simulink model "Complete_Model"  (R2023a)
settings: Solver=VariableStepAuto  StartTime=0.0  StopTime=22  MinStep=5e-5  RelTol=1e-3
values from neighbouring files (symbols this model uses):
  Cbus = 80e-3*n_wt_agg*u^2    (= 1.33333333333)    [param.m]
  D = 1e-3    (= 0.001)    [param.m]
  f = 50    [param.m]
  Fs = Vs * sqrt(2/3)/(2*pi*f)    [param.m]
  fsw = 4e3    (= 4000)    [param.m]
  Idr_rated = sqrt(2)*Is    [param.m]
  J = 127*n_wt_agg    (= 19050)    [param.m]
  ki_id = (wni^2)*Lr*sigma    (= 3.27733001332)    [param.m]
  ki_idg = (wnig^2)*Lg    (= 0.263189450696)    [param.m]
  ki_iq = ki_id    (= 3.27733001332)    [param.m]
  ki_iqg = ki_idg    (= 0.263189450696)    [param.m]
  ki_v = -300000*n_wt_agg    (= -45000000)    [param.m]
  Kopt = ((0.5*rho*pi*(Radius^5)*Cp_max)/(lambda_opt^3))    (= 296454.198186)    [param.m]
  kp_id = (2*wni*sigma*Lr) - Rr    (= 0.00384733333333)    [param.m]
  kp_idg = ((2*wnig*Lg)-Rg)    (= 0.00167538274858)    [param.m]
  kp_iq = kp_id    (= 0.00384733333333)    [param.m]
  kp_iqg = kp_idg    (= 0.00167538274858)    [param.m]
  kp_v = -1000*n_wt_agg    (= -150000)    [param.m]
  Kpg = 1/(1.5*Vr*sqrt(2/3))    [param.m]
  Kqg = -Kpg    [param.m]
  lambda_opt = 7.2    [param.m]
  Lg = 400e-6 /n_wt_agg    (= 2.66666666667e-06)    [param.m]
  Lm = 2.5e-3 /n_wt_agg    (= 1.66666666667e-05)    [param.m]
  Lr = Lm + Lsi    (= 1.72466666667e-05)    [param.m]
  Ls = Lm + Lsi    (= 1.72466666667e-05)    [param.m]
  Lsi = 0.087e-3 /n_wt_agg    (= 5.8e-07)    [param.m]
  N = 100    [param.m]
  n_wt_agg = 150    [param.m]
  p = 2    [param.m]
  Ps = 2e6*n_wt_agg    (= 300000000)    [param.m]
  Radius = 42    [param.m]
  Rg = 20e-6 /n_wt_agg    (= 1.33333333333e-07)    [param.m]
  rho = 1.225    [param.m]
  Rr = 2.9e-3 /n_wt_agg    (= 1.93333333333e-05)    [param.m]
  Rs = 2.6e-3 /n_wt_agg    (= 1.73333333333e-05)    [param.m]
  sigma = 1 - Lm^2 / (Ls*Lr)    (= 0.0661284179513)    [param.m]
  smax = 1/3    (= 0.333333333333)    [param.m]
  tab_lambda = [0.1:0.01:11.8]    [param.m]
  Vbus = 1150/u    (= 3450)    [param.m]
  Vs = 690    [param.m]
undefined symbols (not defined in the file or in its folder): Cp, D1, Delta, DiscIntNeverNeededParam, magnitude
libraries: spsSecondOrderFilterLib x133, spsThreePhaseVIMeasurementLib x58, spsPower3phInstantaneousLib x52, simulink x41, spsThreePhaseTransformerTwoWindingsLib x27, spsUniversalBridgeLib x22, spsPWMGenerator2LevelLib x22, spsSeriesRLCBranchLib x19, spsFirstOrderFilterLib x18, spsThreePhasePISectionLineLib x17, spsGroundLib x17, spsThreePhaseSeriesRLCBranchLib x17
(part 10 of 15)

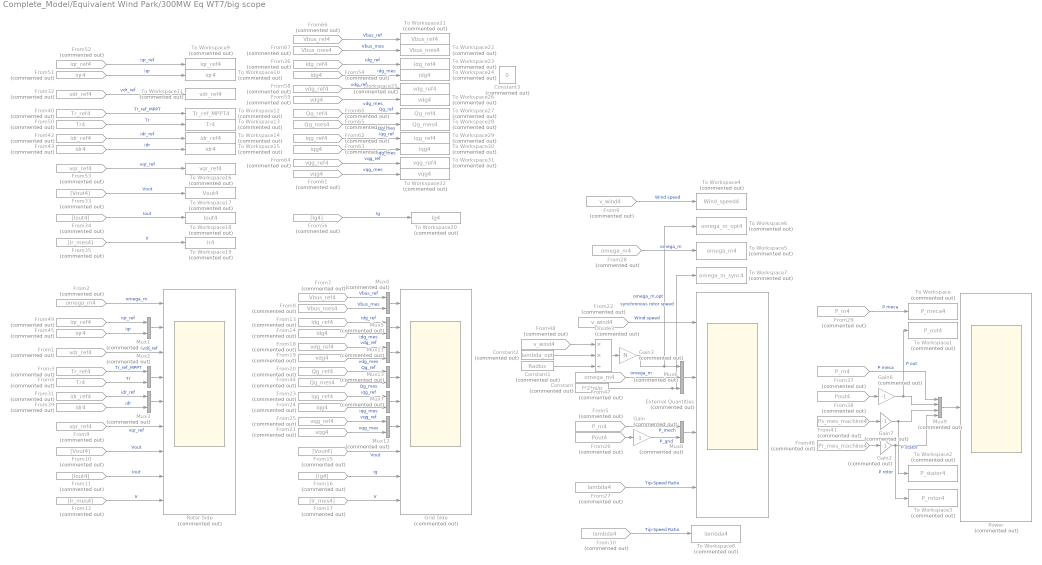
== system Complete_Model/Equivalent Wind Park/300MW Eq WT7/big scope ==
  Constant : Constant  Commented=on  Value=f*2*pi/p
  Constant1 : Constant  Commented=on  Value=Radius
  Constant2 : Constant  Commented=on  Value=lambda_opt
  Constant3 : Constant  Commented=on  Value=0
  Divide3 : Product  Commented=on  Inputs=**/
  External Quantities : Scope  Commented=on  NumInputPorts=4
  From1 : From  Commented=on  GotoTag=vdr_ref4  TagVisibility=global
  From10 : From  Commented=on  GotoTag=Vout4  TagVisibility=global
  From11 : From  Commented=on  GotoTag=Iout4  TagVisibility=global
  From12 : From  Commented=on  GotoTag=Ir_mes4  TagVisibility=global
  From13 : From  Commented=on  GotoTag=idg_ref4  TagVisibility=global
  From14 : From  Commented=on  GotoTag=idg4  TagVisibility=global
  From15 : From  Commented=on  GotoTag=Vout4  TagVisibility=global
  From16 : From  Commented=on  GotoTag=Ig4  TagVisibility=global
  From17 : From  Commented=on  GotoTag=Ir_mes4  TagVisibility=global
  From18 : From  Commented=on  GotoTag=vdg_ref4  TagVisibility=global
  From19 : From  Commented=on  GotoTag=vdg4  TagVisibility=global
  From2 : From  Commented=on  GotoTag=omega_m4  TagVisibility=global
  From20 : From  Commented=on  GotoTag=Qg_ref4  TagVisibility=global
  From21 : From  Commented=on  GotoTag=vqg4  TagVisibility=global
  From22 : From  Commented=on  GotoTag=v_wind4  TagVisibility=global
  From23 : From  Commented=on  GotoTag=iqg_ref4  TagVisibility=global
  From24 : From  Commented=on  GotoTag=iqg4  TagVisibility=global
  From25 : From  Commented=on  GotoTag=vqg_ref4  TagVisibility=global
  From26 : From  Commented=on  GotoTag=Pout4  TagVisibility=global
  From27 : From  Commented=on  GotoTag=lambda4  TagVisibility=global
  From28 : From  Commented=on  GotoTag=omega_m4  TagVisibility=global
  From29 : From  Commented=on  GotoTag=P_m4  TagVisibility=global
  From3 : From  Commented=on  GotoTag=Tr_ref4  TagVisibility=global
  From30 : From  Commented=on  GotoTag=lambda4  TagVisibility=global
  From31 : From  Commented=on  GotoTag=idr_ref4  TagVisibility=global
  From32 : From  Commented=on  GotoTag=vdr_ref4  TagVisibility=global
  From33 : From  Commented=on  GotoTag=Vout4  TagVisibility=global
  From34 : From  Commented=on  GotoTag=Iout4  TagVisibility=global
  From35 : From  Commented=on  GotoTag=Ir_mes4  TagVisibility=global
  From36 : From  Commented=on  GotoTag=idg_ref4  TagVisibility=global
  From37 : From  Commented=on  GotoTag=P_m4  TagVisibility=global
  From38 : From  Commented=on  GotoTag=Pout4  TagVisibility=global
  From39 : From  Commented=on  GotoTag=idr4  TagVisibility=global
  From4 : From  Commented=on  GotoTag=Tr4  TagVisibility=global
  From40 : From  Commented=on  GotoTag=Tr_ref4  TagVisibility=global
  From41 : From  Commented=on  GotoTag=Ps_mes_machine4  TagVisibility=global
  From42 : From  Commented=on  GotoTag=idr_ref4  TagVisibility=global
  From43 : From  Commented=on  GotoTag=idr4  TagVisibility=global
  From44 : From  Commented=on  GotoTag=Qg_mes4  TagVisibility=global
  From45 : From  Commented=on  GotoTag=iqr4  TagVisibility=global
  From46 : From  Commented=on  GotoTag=Pr_mes_machine4  TagVisibility=global
  From47 : From  Commented=on  GotoTag=omega_m4  TagVisibility=global
  From48 : From  Commented=on  GotoTag=v_wind4  TagVisibility=global
  From49 : From  Commented=on  GotoTag=iqr_ref4  TagVisibility=global
  From5 : From  Commented=on  GotoTag=P_m4  TagVisibility=global
  From50 : From  Commented=on  GotoTag=Tr4  TagVisibility=global
  From51 : From  Commented=on  GotoTag=iqr4  TagVisibility=global
  From52 : From  Commented=on  GotoTag=iqr_ref4  TagVisibility=global
  From53 : From  Commented=on  GotoTag=vqr_ref4  TagVisibility=global
  From54 : From  Commented=on  GotoTag=idg4  TagVisibility=global
  From56 : From  Commented=on  GotoTag=Ig4  TagVisibility=global
  From58 : From  Commented=on  GotoTag=vdg_ref4  TagVisibility=global
  From59 : From  Commented=on  GotoTag=vdg4  TagVisibility=global
  From6 : From  Commented=on  GotoTag=v_wind4  TagVisibility=global
  From60 : From  Commented=on  GotoTag=Qg_ref4  TagVisibility=global
  From61 : From  Commented=on  GotoTag=vqg4  TagVisibility=global
  From62 : From  Commented=on  GotoTag=iqg_ref4  TagVisibility=global
  From63 : From  Commented=on  GotoTag=iqg4  TagVisibility=global
  From64 : From  Commented=on  GotoTag=vqg_ref4  TagVisibility=global
  From65 : From  Commented=on  GotoTag=Qg_mes4  TagVisibility=global
  From66 : From  Commented=on  GotoTag=Vbus_ref4  TagVisibility=global
  From67 : From  Commented=on  GotoTag=Vbus_mes4  TagVisibility=global
  From7 : From  Commented=on  GotoTag=Vbus_ref4  TagVisibility=global
  From8 : From  Commented=on  GotoTag=Vbus_mes4  TagVisibility=global
  From9 : From  Commented=on  GotoTag=vqr_ref4  TagVisibility=global
  Gain{,2,6..7} (4 alike) : Gain  Commented=on  Gain=-1
  Gain3 : Gain  Commented=on  Gain=N
  Grid Side : Scope  Commented=on  NumInputPorts=9
  Mux{1..5,7..8,11..13} (10 alike) : Mux  Commented=on  Inputs=2
  Mux6 : Mux  Commented=on  Inputs=3
  Mux9 : Mux  Commented=on
  Power : Scope  Commented=on
  Rotor Side : Scope  Commented=on  NumInputPorts=9
  To Workspace : ToWorkspace  Commented=on  VariableName=P_meca4  SaveFormat=Timeseries  SampleTime=-1
  To Workspace1 : ToWorkspace  Commented=on  VariableName=P_out4  SaveFormat=Timeseries  SampleTime=-1
  To Workspace10 : ToWorkspace  Commented=on  VariableName=iqr4  SaveFormat=Timeseries  SampleTime=-1
  To Workspace11 : ToWorkspace  Commented=on  VariableName=vdr_ref4  SaveFormat=Timeseries  SampleTime=-1
  To Workspace12 : ToWorkspace  Commented=on  VariableName=Tr_ref_MPPT4  SaveFormat=Timeseries  SampleTime=-1
  To Workspace13 : ToWorkspace  Commented=on  VariableName=Tr4  SaveFormat=Timeseries  SampleTime=-1
  To Workspace14 : ToWorkspace  Commented=on  VariableName=idr_ref4  SaveFormat=Timeseries  SampleTime=-1
  To Workspace15 : ToWorkspace  Commented=on  VariableName=idr4  SaveFormat=Timeseries  SampleTime=-1
  To Workspace16 : ToWorkspace  Commented=on  VariableName=vqr_ref4  SaveFormat=Timeseries  SampleTime=-1
  To Workspace17 : ToWorkspace  Commented=on  VariableName=Vout4  SaveFormat=Timeseries  SampleTime=-1
  To Workspace18 : ToWorkspace  Commented=on  VariableName=Iout4  SaveFormat=Timeseries  SampleTime=-1
  To Workspace19 : ToWorkspace  Commented=on  VariableName=Ir4  SaveFormat=Timeseries  SampleTime=-1
  To Workspace2 : ToWorkspace  Commented=on  VariableName=P_stator4  SaveFormat=Timeseries  SampleTime=-1
  To Workspace20 : ToWorkspace  Commented=on  VariableName=Ig4  SaveFormat=Timeseries  SampleTime=-1
  To Workspace21 : ToWorkspace  Commented=on  VariableName=Vbus_ref4  SaveFormat=Timeseries  SampleTime=-1
  To Workspace22 : ToWorkspace  Commented=on  VariableName=Vbus_mes4  SaveFormat=Timeseries  SampleTime=-1
  To Workspace23 : ToWorkspace  Commented=on  VariableName=idg_ref4  SaveFormat=Timeseries  SampleTime=-1
  To Workspace24 : ToWorkspace  Commented=on  VariableName=idg4  SaveFormat=Timeseries  SampleTime=-1
  To Workspace25 : ToWorkspace  Commented=on  VariableName=vdg_ref4  SaveFormat=Timeseries  SampleTime=-1
  To Workspace26 : ToWorkspace  Commented=on  VariableName=vdg4  SaveFormat=Timeseries  SampleTime=-1
  To Workspace27 : ToWorkspace  Commented=on  VariableName=Qg_ref4  SaveFormat=Timeseries  SampleTime=-1
  To Workspace28 : ToWorkspace  Commented=on  VariableName=Qg_mes4  SaveFormat=Timeseries  SampleTime=-1
  To Workspace29 : ToWorkspace  Commented=on  VariableName=iqg_ref4  SaveFormat=Timeseries  SampleTime=-1
  To Workspace3 : ToWorkspace  Commented=on  VariableName=P_rotor4  SaveFormat=Timeseries  SampleTime=-1
  To Workspace30 : ToWorkspace  Commented=on  VariableName=iqg4  SaveFormat=Timeseries  SampleTime=-1
  To Workspace31 : ToWorkspace  Commented=on  VariableName=vqg_ref4  SaveFormat=Timeseries  SampleTime=-1
  To Workspace32 : ToWorkspace  Commented=on  VariableName=vqg4  SaveFormat=Timeseries  SampleTime=-1
  To Workspace4 : ToWorkspace  Commented=on  VariableName=Wind_speed4  SaveFormat=Timeseries  SampleTime=-1
  To Workspace5 : ToWorkspace  Commented=on  VariableName=omega_m4  SaveFormat=Timeseries  SampleTime=-1
  To Workspace6 : ToWorkspace  Commented=on  VariableName=omega_m_opt4  SaveFormat=Timeseries  SampleTime=-1
  To Workspace7 : ToWorkspace  Commented=on  VariableName=omega_m_sync4  SaveFormat=Timeseries  SampleTime=-1
  To Workspace8 : ToWorkspace  Commented=on  VariableName=lambda4  SaveFormat=Timeseries  SampleTime=-1
  To Workspace9 : ToWorkspace  Commented=on  VariableName=iqr_ref4  SaveFormat=Timeseries  SampleTime=-1
  wires:
  From61/1 -> To Workspace32/1  "vqg_mes"
  From64/1 -> To Workspace31/1  "vqg_ref"
  From63/1 -> To Workspace30/1  "iqg_mes"
  From62/1 -> To Workspace29/1  "iqg_ref"
  From65/1 -> To Workspace28/1  "Qg_mes"
  From59/1 -> To Workspace26/1  "vdg_mes"
  From60/1 -> To Workspace27/1  "Qg_ref"
  From58/1 -> To Workspace25/1  "vdg_ref"
  From54/1 -> To Workspace24/1  "idg_mes"
  From36/1 -> To Workspace23/1  "idg_ref"
  From67/1 -> To Workspace22/1  "Vbus_mes"
  From66/1 -> To Workspace21/1  "Vbus_ref"
  From56/1 -> To Workspace20/1  "Ig"
  From35/1 -> To Workspace19/1  "Ir"
  From34/1 -> To Workspace18/1  "Iout"
  From33/1 -> To Workspace17/1  "Vout"
  From53/1 -> To Workspace16/1  "vqr_ref"
  From43/1 -> To Workspace15/1  "idr"
  From42/1 -> To Workspace14/1  "idr_ref"
  From50/1 -> To Workspace13/1  "Tr"
  From40/1 -> To Workspace12/1  "Tr_ref_MPPT"
  From32/1 -> To Workspace11/1  "vdr_ref"
  From51/1 -> To Workspace10/1  "iqr"
  From52/1 -> To Workspace9/1  "iqr_ref"
  From30/1 -> To Workspace8/1  "Tip-Speed Ratio"
  From29/1 -> To Workspace/1  "P meca"
  From28/1 -> To Workspace5/1  "omega_m"
  From6/1 -> To Workspace4/1  "Wind speed"
  From27/1 -> External Quantities/4  "Tip-Speed Ratio"
  Gain3/1 -> Mux6/1, To Workspace6/1  "omega_m,opt"
  Divide3/1 -> Gain3/1
  Constant1/1 -> Divide3/3
  Constant2/1 -> Divide3/2
  From48/1 -> Divide3/1
  From39/1 -> Mux3/2  "idr"
  From4/1 -> Mux2/2  "Tr"
  From1/1 -> Rotor Side/3  "vdr_ref"
  From49/1 -> Mux1/1  "iqr_ref"
  Mux13/1 -> Grid Side/4
  From44/1 -> Mux13/2  "Qg_mes"
  From20/1 -> Mux13/1  "Qg_ref"
  Mux12/1 -> Grid Side/6
  From25/1 -> Mux12/1  "vqg_ref"
  From21/1 -> Mux12/2  "vqg_mes"
  Gain2/1 -> Mux9/4, To Workspace3/1  "P rotor"
  From38/1 -> Gain6/1
  Mux9/1 -> Power/1
  From37/1 -> Mux9/1  "P meca"
  From46/1 -> Gain2/1
  Gain7/1 -> Mux9/3, To Workspace2/1  "P stator"
  From41/1 -> Gain7/1
  Gain6/1 -> Mux9/2, To Workspace1/1  "P out"
  Mux11/1 -> Grid Side/3
  From19/1 -> Mux11/2  "vdg_mes"
  From18/1 -> Mux11/1  "vdg_ref"
  From5/1 -> Mux8/1  "P_mech"
  Constant/1 -> Mux6/3, To Workspace7/1  "synchronous rotor speed"
  From47/1 -> Mux6/2  "omega_m"
  From22/1 -> External Quantities/1  "Wind speed"
  Mux6/1 -> External Quantities/2
  Mux8/1 -> External Quantities/3
  From26/1 -> Gain/1
  Gain/1 -> Mux8/2  "P_grid"
  From2/1 -> Rotor Side/1  "omega_m"
  From13/1 -> Mux5/1  "idg_ref"
  Mux4/1 -> Grid Side/1
  From14/1 -> Mux5/2  "idg_mes"
  Mux5/1 -> Grid Side/2
  From8/1 -> Mux4/2  "Vbus_mes"
  Mux7/1 -> Grid Side/5
  From7/1 -> Mux4/1  "Vbus_ref"
  From24/1 -> Mux7/2  "iqg_mes"
  From17/1 -> Grid Side/9  "Ir"
  From15/1 -> Grid Side/7  "Vout"
  From23/1 -> Mux7/1  "iqg_ref"
  From16/1 -> Grid Side/8  "Ig"
  From12/1 -> Rotor Side/9  "Ir"
  From11/1 -> Rotor Side/8  "Iout"
  From10/1 -> Rotor Side/7  "Vout"
  From9/1 -> Rotor Side/6  "vqr_ref"
  From31/1 -> Mux3/1  "idr_ref"
  Mux3/1 -> Rotor Side/5
  Mux2/1 -> Rotor Side/4
  From3/1 -> Mux2/1  "Tr_ref_MPPT"
  Mux1/1 -> Rotor Side/2
  From45/1 -> Mux1/2  "iqr"

== system Complete_Model/Equivalent Wind Park/300MW Eq WT8/Aerodynamic Model ==
  note: Gearbox ratio
  note: Motor convention : Generated power
      |  is negative
  note: \Omega_m blades
  note: Cp
  note: \lambda = \Omega_m R / v_{wind}
  note: C_p (\lambda)
  note: v_{wind}
  Vw : Inport
  C_p (\lambda) : Lookup_n-D  NumberOfTableDimensions=1  BreakpointsForDimension1=tab_lambda  Table=Cp  InternalRulePriority=Speed  RndMeth=Simplest
  Constant : Constant  Value=Radius
  Divide : Product  Inputs=*/
  Divide1 : Product  Inputs=**/
  Fcn2 : Fcn  Expr=0.5*rho*pi*Radius^2*u(2)^3*u(1)
  Gain : Gain  Gain=1/N
  Gain2 : Gain  Gain=n_wt_agg
  Gain4 : Gain  Gain=-1
  Mux2 : Mux  Inputs=2
  Tm : Outport
  tag scope: P_m11=global, Tm11=global, lambda11=global
  wires:
  Gain4/1 -> Divide/1
  Constant/1 -> Divide1/1
  Divide/1 -> Tm/1, [Tm11]
  [omega_m11] -> Divide/2
  Gain2/1 -> Gain4/1, [P_m11]
  Fcn2/1 -> Gain2/1
  Mux2/1 -> Fcn2/1
  C_p (\lambda)/1 -> Mux2/1
  Divide1/1 -> [lambda11], C_p (\lambda)/1  "lambda"
  Vw/1 -> Mux2/2, Divide1/3
  Gain/1 -> Divide1/2
  [omega_m11] -> Gain/1

== system Complete_Model/Equivalent Wind Park/300MW Eq WT8/Grid Side Control ==
  Vbus_ref : Inport
  Qg_ref : Inport  Port=2
  Vbus : Inport  Port=3
  Ig_mes : Inport  Port=4
  Vs_mes : Inport  Port=5
  Demux : Demux  Outputs=2
  Demux1 : Demux  Outputs=2
  Gain : Gain  Gain=Kqg
  Gain1 : Gain  Gain=Kpg
  Normalized for PWM : Gain  Gain=1/(Vbus/2)
  "PI " : Subsystem
  PI id : Subsystem
  PI iq : Subsystem
  Sum : Sum  Inputs=|++
  Vs_mes1 : InportShadow  Port=5
  abc->alpha/beta : Subsystem
  abc->alpha/beta1 : Subsystem
  alpha/beta->abc : Subsystem
  alpha/beta->d/q : Subsystem
  alpha/beta->d/q1 : Subsystem
  angle2 : Subsystem
  cancellation of coupling terms : Subsystem
  d/q->alpha/beta : Subsystem
  Vabc_ref : Outport
  tag scope: Qg_ref11=global, Vbus_ref11=global, idg11=global, idg_ref11=global, iqg11=global, iqg_ref11=global, vdg11=global, vqg11=global
  wires:
  Vs_mes1/1 -> Sum/2
  angle2/1(theta_g) -> [theta_grid11]
  [theta_grid11] -> alpha/beta->d/q1/2(theta)
  [theta_grid11] -> d/q->alpha/beta/2(theta)
  [theta_grid11] -> alpha/beta->d/q/2(theta)
  Demux/2 -> PI iq/2(meas), [iqg11]
  Demux/1 -> PI id/2(meas), [idg11]
  Vbus/1 -> "PI "/2(meas)
  Demux1/1 -> [vdg11]
  Demux1/2 -> [vqg11]
  alpha/beta->d/q1/1(d/q) -> Demux1/1
  abc->alpha/beta1/1(alpha/beta) -> alpha/beta->d/q1/1(D/Q)
  [Vg11] -> abc->alpha/beta1/1(abc)
  cancellation of coupling terms/1(out) -> d/q->alpha/beta/1(d/q)
  Sum/1 -> Normalized for PWM/1
  alpha/beta->abc/1(abc) -> Sum/1
  Qg_ref/1 -> Gain/1, [Qg_ref11]
  Gain1/1 -> PI id/1(ref), [idg_ref11]  "idg"
  "PI "/1(out) -> Gain1/1  "Pg_ref"
  Gain/1 -> [iqg_ref11], PI iq/1(ref)  "iqg"
  Vbus_ref/1 -> "PI "/1(ref), [Vbus_ref11]
  alpha/beta->d/q/1(d/q) -> Demux/1
  PI iq/1(out) -> cancellation of coupling terms/2(Vq*)
  PI id/1(out) -> cancellation of coupling terms/1(Vd*)
  Vs_mes/1 -> angle2/1(Vs_mes)
  Normalized for PWM/1 -> Vabc_ref/1
  abc->alpha/beta/1(alpha/beta) -> alpha/beta->d/q/1(D/Q)
  Ig_mes/1 -> abc->alpha/beta/1(abc)
  d/q->alpha/beta/1(D/Q) -> alpha/beta->abc/1(D/Q)

== system Complete_Model/Equivalent Wind Park/300MW Eq WT8/Power Calculations ==
  Demux2 : Demux  Outputs=2
  Demux3 : Demux  Outputs=2
  Power (3ph, Instantaneous){1..4} (4 alike) : spsPower3phInstantaneousLib/Power (3ph, Instantaneous)
  Scope{1,3..5,10..13} (8 alike) : Scope
  "Second-Order " : spsSecondOrderFilterLib/Second-Order Filter  FilterType=Lowpass  Fo=17  Zeta=0.7  Ts=7.4074e-05  Initialize=off  Vac_Init="[0,  0,  60]"  Vdc_Init=0  PlotResponse=off  FreqRange=[0, 500, 1]
      settings: AttributesFormatString=f0=%<Fo>
  Second-Order Low-Pass Filter {1..7} (7 alike) : spsSecondOrderFilterLib/Second-Order Filter  FilterType=Lowpass  Fo=17  Zeta=0.7  Ts=7.4074e-05  Initialize=off  Vac_Init="[0,  0,  60]"  Vdc_Init=0  PlotResponse=off  FreqRange=[0, 500, 1]
      settings: AttributesFormatString=f0=%<Fo>
  Subsystem2 : Subsystem
  Subsystem3 : Subsystem
  To Workspace2 : ToWorkspace  VariableName=Ig_d11  SaveFormat=Timeseries  SampleTime=-1
  To Workspace3 : ToWorkspace  VariableName=Vg_d11  SaveFormat=Timeseries  SampleTime=-1
  ToFile_Ig_d11 : ToFile  Filename=Ig_d11.mat  SaveFormat=Timeseries
  ToFile_Vg_d11 : ToFile  Filename=Vg_d11.mat  SaveFormat=Timeseries
  alpha/beta->d/q2 : Subsystem
  alpha/beta->d/q3 : Subsystem
  angle1 : Subsystem
  tag scope: Pg_mes11=global, Pout11=global, Pr_mes_machine11=global, Ps_mes_machine11=global, Qg_mes11=global, Qout11=global, Qr_mes_machine11=global, Qs_mes_machine11=global
  wires:
  [Vg11] -> angle1/1(Vs_mes), Subsystem2/1(abc)
  Subsystem2/1(alpha/beta) -> alpha/beta->d/q2/1(D/Q)
  alpha/beta->d/q2/1(d/q) -> Demux2/1
  Demux2/1 -> ToFile_Vg_d11/1, Scope10/1, To Workspace3/1  "Vg_d11"
  Demux2/2 -> Scope11/1
  Demux3/2 -> Scope13/1
  Demux3/1 -> ToFile_Ig_d11/1, Scope12/1, To Workspace2/1  "Ig_d11"
  alpha/beta->d/q3/1(d/q) -> Demux3/1
  Subsystem3/1(alpha/beta) -> alpha/beta->d/q3/1(D/Q)
  angle1/1(theta_g) -> alpha/beta->d/q3/2(theta), alpha/beta->d/q2/2(theta)
  [Ig11] -> Subsystem3/1(abc)
  [Vr_mes11] -> "Power (3ph, Instantaneous)4"/1
  "Power (3ph, Instantaneous)1"/1 -> Second-Order Low-Pass Filter 7/1  "Pr"
  "Power (3ph, Instantaneous)4"/1 -> Second-Order Low-Pass Filter 5/1  "Pr"
  [Vout11] -> "Power (3ph, Instantaneous)2"/1
  "Power (3ph, Instantaneous)3"/2 -> Second-Order Low-Pass Filter 2/1
  [Vg11] -> "Power (3ph, Instantaneous)1"/1
  Second-Order Low-Pass Filter 2/1 -> [Qs_mes_machine11]  "Q_stator"
  [Vs_mes_machine11] -> "Power (3ph, Instantaneous)3"/1
  "Power (3ph, Instantaneous)2"/1 -> "Second-Order "/1
  Second-Order Low-Pass Filter 5/1 -> [Pr_mes_machine11]  "P_rotor"
  [Is_mes_machine11] -> "Power (3ph, Instantaneous)3"/2, Scope4/1
  Second-Order Low-Pass Filter 7/1 -> [Pg_mes11]  "P_rotor"
  [Ir_mes11] -> "Power (3ph, Instantaneous)4"/2, Scope5/1
  "Second-Order "/1 -> [Pout11]  "Pout"
  Second-Order Low-Pass Filter 6/1 -> [Qg_mes11]  "Q_rotor"
  Second-Order Low-Pass Filter 4/1 -> [Qr_mes_machine11]  "Q_rotor"
  "Power (3ph, Instantaneous)3"/1 -> Second-Order Low-Pass Filter 3/1  "Ps"
  [Ig11] -> Scope1/1, "Power (3ph, Instantaneous)1"/2
  "Power (3ph, Instantaneous)4"/2 -> Second-Order Low-Pass Filter 4/1
  [Iout11] -> "Power (3ph, Instantaneous)2"/2, Scope3/1
  "Power (3ph, Instantaneous)1"/2 -> Second-Order Low-Pass Filter 6/1
  Second-Order Low-Pass Filter 3/1 -> [Ps_mes_machine11]  "P_stator"
  Second-Order Low-Pass Filter 1/1 -> [Qout11]  "Qout"
  "Power (3ph, Instantaneous)2"/2 -> Second-Order Low-Pass Filter 1/1

== system Complete_Model/Equivalent Wind Park/300MW Eq WT8/Rotor Side Control ==
  note: Stator referred
  idr_ref : Inport
  Ir_mes : Inport  Port=2
  Vs_mes : Inport  Port=3
  theta_m_mes : Inport  Port=4
  speed_mes : Inport  Port=5
  D/Q->abc : Subsystem
  D/Q->d/q : Subsystem
  Demux : Demux  Outputs=2
  Gain : Gain  Gain=1/(-1.5*p*(Lm/Ls)*Fs)
  Gain1 : Gain  Gain=1/u
  Gain2 : Gain  Gain=1/u
  MPPT : Subsystem
  Normalized for PWM : Gain  Gain=1/(Vbus/2)
  PI id : Subsystem
  PI iq : Subsystem
  abc->alpha/beta : Subsystem
  angle : Subsystem
  cancellation of coupling terms : Subsystem
  d/q->D/Q : Subsystem
  Vabc_ref : Outport
  tag scope: Tr_ref11=global, idr11=global, idr_ref11=global, iqr11=global, iqr_ref11=global, vrabc_ref11=global
  wires:
  MPPT/1(Tm,opt) -> Gain/1, [Tr_ref11]
  d/q->D/Q/1(D/Q) -> D/Q->abc/1(D/Q)
  abc->alpha/beta/1(alpha/beta) -> D/Q->d/q/1(D/Q)
  Gain2/1 -> [vrabc_ref11], Normalized for PWM/1  "Rotor referred"
  D/Q->abc/1(abc) -> Gain2/1
  Normalized for PWM/1 -> Vabc_ref/1
  Gain1/1 -> abc->alpha/beta/1(abc)
  idr_ref/1 -> PI id/1(ref), [idr_ref11]
  Gain/1 -> PI iq/1(ref), [iqr_ref11]
  Demux/2 -> PI iq/2(meas), [iqr11]
  Demux/1 -> [idr11], PI id/2(meas)
  D/Q->d/q/1(d/q) -> Demux/1  "i_dq = rotor ref frame"
  speed_mes/1 -> MPPT/1(omega_m), cancellation of coupling terms/3(omega_m)
  cancellation of coupling terms/1(out) -> d/q->D/Q/1(d/q)  "Vdr_ref,Vqr_ref"
  PI iq/1(out) -> cancellation of coupling terms/2(Vq*)
  PI id/1(out) -> cancellation of coupling terms/1(Vd*)
  angle/1(theta_r) -> D/Q->d/q/2(theta), d/q->D/Q/2(theta)
  Vs_mes/1 -> angle/1(Vs_mes)
  theta_m_mes/1 -> angle/2(theta_m)
  Ir_mes/1 -> Gain1/1

== system Complete_Model/Equivalent Wind Park/300MW Eq WT8/Subsystem ==
  In1 : Inport
  Bus Selector : BusSelector  OutputSignals=Mechanical.Rotor angle thetam (rad),Mechanical.Rotor speed (wm),Mechanical.Electromagnetic torque Te (N*m)
  Second-Order Low-Pass Filter 3 : spsSecondOrderFilterLib/Second-Order Filter  FilterType=Lowpass  Fo=17  Zeta=0.7  Ts=7.4074e-05  Initialize=off  Vac_Init="[0,  0,  60]"  Vdc_Init=0  PlotResponse=off  FreqRange=[0, 500, 1]
      settings: AttributesFormatString=f0=%<Fo>
  tag scope: Tr11=global, omega_m11=global, theta11=global
  wires:
  Second-Order Low-Pass Filter 3/1 -> [omega_m11]
  Bus Selector/3 -> [Tr11]  "<Electromagnetic torque Te (N*m)>"
  Bus Selector/1 -> [theta11]  "<Rotor angle thetam (rad)>"
  In1/1 -> Bus Selector/1
  Bus Selector/2 -> Second-Order Low-Pass Filter 3/1  "<Rotor speed (wm)>"

== system Complete_Model/Equivalent Wind Park/300MW Eq WT8/Subsystem1 ==
  Constant4 : Constant  Value=0
  Step : Step  Time=50  After=-0.2*Ps  SampleTime=0
  Sum14 : Sum  Inputs=++|
  Out1 : Outport
  wires:
  Sum14/1 -> Out1/1
  Step/1 -> Sum14/1
  Constant4/1 -> Sum14/2

== system Complete_Model/Equivalent Wind Park/300MW Eq WT8/Subsystem2 ==
  Constant1 : Constant  Commented=on  Value=8
  Constant2 : Constant  Value=9
  Ramp1 : simulink/Sources/Ramp  Commented=on  slope=-1  start=16  InitialOutput=0
  Ramp2 : simulink/Sources/Ramp  Commented=on  slope=1  start=13  InitialOutput=0
  Ramp3 : simulink/Sources/Ramp  Commented=on  slope=-1  start=36  InitialOutput=0
  Ramp4 : simulink/Sources/Ramp  Commented=on  slope=1  start=35  InitialOutput=0
  Ramp5 : simulink/Sources/Ramp  slope=-0.4  start=13  InitialOutput=0
  Ramp6 : simulink/Sources/Ramp  slope=0.4  start=8  InitialOutput=0
  Sum{1..4} (4 alike) : Sum  Commented=on  Inputs=++|
  Sum5 : Sum  Inputs=++|
  Sum6 : Sum  Inputs=++|
  v_wind : Outport
  tag scope: v_wind11=global
  wires:
  Sum4/1 -> ?
  Ramp2/1 -> Sum1/1
  Ramp1/1 -> Sum1/2
  Sum1/1 -> Sum2/1
  Sum3/1 -> Sum4/1
  Ramp3/1 -> Sum3/2
  Ramp4/1 -> Sum3/1
  Sum2/1 -> Sum4/2
  Constant1/1 -> Sum2/2
  Ramp5/1 -> Sum5/2
  Constant2/1 -> Sum6/2
  Ramp6/1 -> Sum5/1
  Sum5/1 -> Sum6/1
  Sum6/1 -> v_wind/1, [v_wind11]

== system Complete_Model/Equivalent Wind Park/300MW Eq WT8/Subsystem8 ==
  Constant4 : Constant  Value=0
  Step : Step  Time=55  After=Idr_rated*0.2  SampleTime=0
  Sum14 : Sum  Inputs=++|
  Out1 : Outport
  wires:
  Sum14/1 -> Out1/1
  Constant4/1 -> Sum14/2
  Step/1 -> Sum14/1

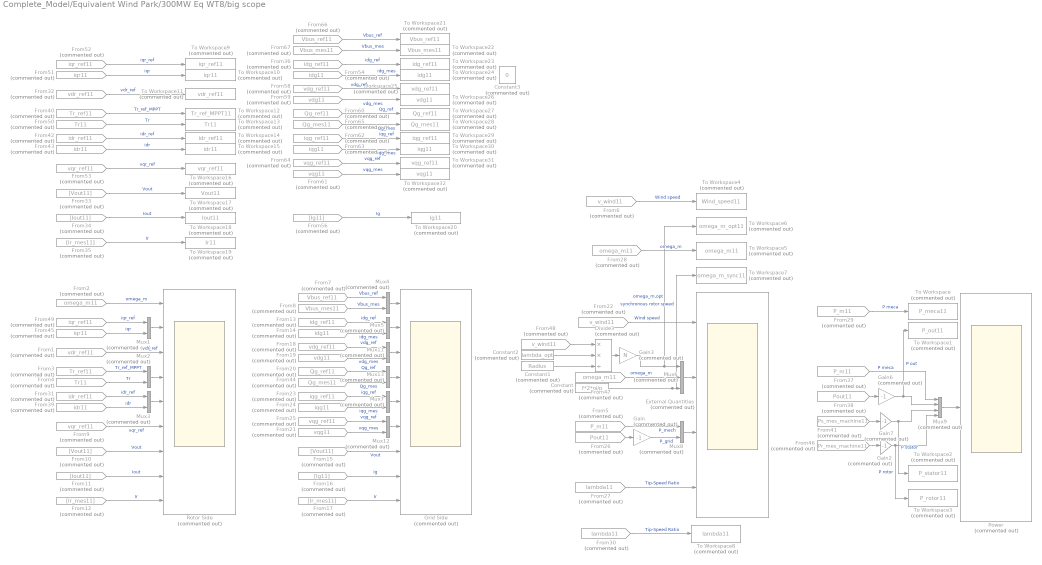
== system Complete_Model/Equivalent Wind Park/300MW Eq WT8/big scope ==
  Constant : Constant  Commented=on  Value=f*2*pi/p
  Constant1 : Constant  Commented=on  Value=Radius
  Constant2 : Constant  Commented=on  Value=lambda_opt
  Constant3 : Constant  Commented=on  Value=0
  Divide3 : Product  Commented=on  Inputs=**/
  External Quantities : Scope  Commented=on  NumInputPorts=4
  From1 : From  Commented=on  GotoTag=vdr_ref11  TagVisibility=global
  From10 : From  Commented=on  GotoTag=Vout11  TagVisibility=global
  From11 : From  Commented=on  GotoTag=Iout11  TagVisibility=global
  From12 : From  Commented=on  GotoTag=Ir_mes11  TagVisibility=global
  From13 : From  Commented=on  GotoTag=idg_ref11  TagVisibility=global
  From14 : From  Commented=on  GotoTag=idg11  TagVisibility=global
  From15 : From  Commented=on  GotoTag=Vout11  TagVisibility=global
  From16 : From  Commented=on  GotoTag=Ig11  TagVisibility=global
  From17 : From  Commented=on  GotoTag=Ir_mes11  TagVisibility=global
  From18 : From  Commented=on  GotoTag=vdg_ref11  TagVisibility=global
  From19 : From  Commented=on  GotoTag=vdg11  TagVisibility=global
  From2 : From  Commented=on  GotoTag=omega_m11  TagVisibility=global
  From20 : From  Commented=on  GotoTag=Qg_ref11  TagVisibility=global
  From21 : From  Commented=on  GotoTag=vqg11  TagVisibility=global
  From22 : From  Commented=on  GotoTag=v_wind11  TagVisibility=global
  From23 : From  Commented=on  GotoTag=iqg_ref11  TagVisibility=global
  From24 : From  Commented=on  GotoTag=iqg11  TagVisibility=global
  From25 : From  Commented=on  GotoTag=vqg_ref11  TagVisibility=global
  From26 : From  Commented=on  GotoTag=Pout11  TagVisibility=global
  From27 : From  Commented=on  GotoTag=lambda11  TagVisibility=global
  From28 : From  Commented=on  GotoTag=omega_m11  TagVisibility=global
  From29 : From  Commented=on  GotoTag=P_m11  TagVisibility=global
  From3 : From  Commented=on  GotoTag=Tr_ref11  TagVisibility=global
  From30 : From  Commented=on  GotoTag=lambda11  TagVisibility=global
  From31 : From  Commented=on  GotoTag=idr_ref11  TagVisibility=global
  From32 : From  Commented=on  GotoTag=vdr_ref11  TagVisibility=global
  From33 : From  Commented=on  GotoTag=Vout11  TagVisibility=global
  From34 : From  Commented=on  GotoTag=Iout11  TagVisibility=global
  From35 : From  Commented=on  GotoTag=Ir_mes11  TagVisibility=global
  From36 : From  Commented=on  GotoTag=idg_ref11  TagVisibility=global
  From37 : From  Commented=on  GotoTag=P_m11  TagVisibility=global
  From38 : From  Commented=on  GotoTag=Pout11  TagVisibility=global
  From39 : From  Commented=on  GotoTag=idr11  TagVisibility=global
  From4 : From  Commented=on  GotoTag=Tr11  TagVisibility=global
  From40 : From  Commented=on  GotoTag=Tr_ref11  TagVisibility=global
  From41 : From  Commented=on  GotoTag=Ps_mes_machine11  TagVisibility=global
  From42 : From  Commented=on  GotoTag=idr_ref11  TagVisibility=global
  From43 : From  Commented=on  GotoTag=idr11  TagVisibility=global
  From44 : From  Commented=on  GotoTag=Qg_mes11  TagVisibility=global
  From45 : From  Commented=on  GotoTag=iqr11  TagVisibility=global
  From46 : From  Commented=on  GotoTag=Pr_mes_machine11  TagVisibility=global
  From47 : From  Commented=on  GotoTag=omega_m11  TagVisibility=global
  From48 : From  Commented=on  GotoTag=v_wind11  TagVisibility=global
  From49 : From  Commented=on  GotoTag=iqr_ref11  TagVisibility=global
  From5 : From  Commented=on  GotoTag=P_m11  TagVisibility=global
  From50 : From  Commented=on  GotoTag=Tr11  TagVisibility=global
  From51 : From  Commented=on  GotoTag=iqr11  TagVisibility=global
  From52 : From  Commented=on  GotoTag=iqr_ref11  TagVisibility=global
  From53 : From  Commented=on  GotoTag=vqr_ref11  TagVisibility=global
  From54 : From  Commented=on  GotoTag=idg11  TagVisibility=global
  From56 : From  Commented=on  GotoTag=Ig11  TagVisibility=global
  From58 : From  Commented=on  GotoTag=vdg_ref11  TagVisibility=global
  From59 : From  Commented=on  GotoTag=vdg11  TagVisibility=global
  From6 : From  Commented=on  GotoTag=v_wind11  TagVisibility=global
  From60 : From  Commented=on  GotoTag=Qg_ref11  TagVisibility=global
  From61 : From  Commented=on  GotoTag=vqg11  TagVisibility=global
  From62 : From  Commented=on  GotoTag=iqg_ref11  TagVisibility=global
  From63 : From  Commented=on  GotoTag=iqg11  TagVisibility=global
  From64 : From  Commented=on  GotoTag=vqg_ref11  TagVisibility=global
  From65 : From  Commented=on  GotoTag=Qg_mes11  TagVisibility=global
  From66 : From  Commented=on  GotoTag=Vbus_ref11  TagVisibility=global
  From67 : From  Commented=on  GotoTag=Vbus_mes11  TagVisibility=global
  From7 : From  Commented=on  GotoTag=Vbus_ref11  TagVisibility=global
  From8 : From  Commented=on  GotoTag=Vbus_mes11  TagVisibility=global
  From9 : From  Commented=on  GotoTag=vqr_ref11  TagVisibility=global
  Gain{,2,6..7} (4 alike) : Gain  Commented=on  Gain=-1
  Gain3 : Gain  Commented=on  Gain=N
  Grid Side : Scope  Commented=on  NumInputPorts=9
  Mux{1..5,7..8,11..13} (10 alike) : Mux  Commented=on  Inputs=2
  Mux6 : Mux  Commented=on  Inputs=3
  Mux9 : Mux  Commented=on
  Power : Scope  Commented=on
  Rotor Side : Scope  Commented=on  NumInputPorts=9
  To Workspace : ToWorkspace  Commented=on  VariableName=P_meca11  SaveFormat=Timeseries  SampleTime=-1
  To Workspace1 : ToWorkspace  Commented=on  VariableName=P_out11  SaveFormat=Timeseries  SampleTime=-1
  To Workspace10 : ToWorkspace  Commented=on  VariableName=iqr11  SaveFormat=Timeseries  SampleTime=-1
  To Workspace11 : ToWorkspace  Commented=on  VariableName=vdr_ref11  SaveFormat=Timeseries  SampleTime=-1
  To Workspace12 : ToWorkspace  Commented=on  VariableName=Tr_ref_MPPT11  SaveFormat=Timeseries  SampleTime=-1
  To Workspace13 : ToWorkspace  Commented=on  VariableName=Tr11  SaveFormat=Timeseries  SampleTime=-1
  To Workspace14 : ToWorkspace  Commented=on  VariableName=idr_ref11  SaveFormat=Timeseries  SampleTime=-1
  To Workspace15 : ToWorkspace  Commented=on  VariableName=idr11  SaveFormat=Timeseries  SampleTime=-1
  To Workspace16 : ToWorkspace  Commented=on  VariableName=vqr_ref11  SaveFormat=Timeseries  SampleTime=-1
  To Workspace17 : ToWorkspace  Commented=on  VariableName=Vout11  SaveFormat=Timeseries  SampleTime=-1
  To Workspace18 : ToWorkspace  Commented=on  VariableName=Iout11  SaveFormat=Timeseries  SampleTime=-1
  To Workspace19 : ToWorkspace  Commented=on  VariableName=Ir11  SaveFormat=Timeseries  SampleTime=-1
  To Workspace2 : ToWorkspace  Commented=on  VariableName=P_stator11  SaveFormat=Timeseries  SampleTime=-1
  To Workspace20 : ToWorkspace  Commented=on  VariableName=Ig11  SaveFormat=Timeseries  SampleTime=-1
  To Workspace21 : ToWorkspace  Commented=on  VariableName=Vbus_ref11  SaveFormat=Timeseries  SampleTime=-1
  To Workspace22 : ToWorkspace  Commented=on  VariableName=Vbus_mes11  SaveFormat=Timeseries  SampleTime=-1
  To Workspace23 : ToWorkspace  Commented=on  VariableName=idg_ref11  SaveFormat=Timeseries  SampleTime=-1
  To Workspace24 : ToWorkspace  Commented=on  VariableName=idg11  SaveFormat=Timeseries  SampleTime=-1
  To Workspace25 : ToWorkspace  Commented=on  VariableName=vdg_ref11  SaveFormat=Timeseries  SampleTime=-1
  To Workspace26 : ToWorkspace  Commented=on  VariableName=vdg11  SaveFormat=Timeseries  SampleTime=-1
  To Workspace27 : ToWorkspace  Commented=on  VariableName=Qg_ref11  SaveFormat=Timeseries  SampleTime=-1
  To Workspace28 : ToWorkspace  Commented=on  VariableName=Qg_mes11  SaveFormat=Timeseries  SampleTime=-1
  To Workspace29 : ToWorkspace  Commented=on  VariableName=iqg_ref11  SaveFormat=Timeseries  SampleTime=-1
  To Workspace3 : ToWorkspace  Commented=on  VariableName=P_rotor11  SaveFormat=Timeseries  SampleTime=-1
  To Workspace30 : ToWorkspace  Commented=on  VariableName=iqg11  SaveFormat=Timeseries  SampleTime=-1
  To Workspace31 : ToWorkspace  Commented=on  VariableName=vqg_ref11  SaveFormat=Timeseries  SampleTime=-1
  To Workspace32 : ToWorkspace  Commented=on  VariableName=vqg11  SaveFormat=Timeseries  SampleTime=-1
  To Workspace4 : ToWorkspace  Commented=on  VariableName=Wind_speed11  SaveFormat=Timeseries  SampleTime=-1
  To Workspace5 : ToWorkspace  Commented=on  VariableName=omega_m11  SaveFormat=Timeseries  SampleTime=-1
  To Workspace6 : ToWorkspace  Commented=on  VariableName=omega_m_opt11  SaveFormat=Timeseries  SampleTime=-1
  To Workspace7 : ToWorkspace  Commented=on  VariableName=omega_m_sync11  SaveFormat=Timeseries  SampleTime=-1
  To Workspace8 : ToWorkspace  Commented=on  VariableName=lambda11  SaveFormat=Timeseries  SampleTime=-1
  To Workspace9 : ToWorkspace  Commented=on  VariableName=iqr_ref11  SaveFormat=Timeseries  SampleTime=-1
  wires:
  From61/1 -> To Workspace32/1  "vqg_mes"
  From64/1 -> To Workspace31/1  "vqg_ref"
  From63/1 -> To Workspace30/1  "iqg_mes"
  From62/1 -> To Workspace29/1  "iqg_ref"
  From65/1 -> To Workspace28/1  "Qg_mes"
  From59/1 -> To Workspace26/1  "vdg_mes"
  From60/1 -> To Workspace27/1  "Qg_ref"
  From58/1 -> To Workspace25/1  "vdg_ref"
  From54/1 -> To Workspace24/1  "idg_mes"
  From36/1 -> To Workspace23/1  "idg_ref"
  From67/1 -> To Workspace22/1  "Vbus_mes"
  From66/1 -> To Workspace21/1  "Vbus_ref"
  From56/1 -> To Workspace20/1  "Ig"
  From35/1 -> To Workspace19/1  "Ir"
  From34/1 -> To Workspace18/1  "Iout"
  From33/1 -> To Workspace17/1  "Vout"
  From53/1 -> To Workspace16/1  "vqr_ref"
  From43/1 -> To Workspace15/1  "idr"
  From42/1 -> To Workspace14/1  "idr_ref"
  From50/1 -> To Workspace13/1  "Tr"
  From40/1 -> To Workspace12/1  "Tr_ref_MPPT"
  From32/1 -> To Workspace11/1  "vdr_ref"
  From51/1 -> To Workspace10/1  "iqr"
  From52/1 -> To Workspace9/1  "iqr_ref"
  From30/1 -> To Workspace8/1  "Tip-Speed Ratio"
  From29/1 -> To Workspace/1  "P meca"
  From28/1 -> To Workspace5/1  "omega_m"
  From6/1 -> To Workspace4/1  "Wind speed"
  From27/1 -> External Quantities/4  "Tip-Speed Ratio"
  Gain3/1 -> Mux6/1, To Workspace6/1  "omega_m,opt"
  Divide3/1 -> Gain3/1
  Constant1/1 -> Divide3/3
  Constant2/1 -> Divide3/2
  From48/1 -> Divide3/1
  From39/1 -> Mux3/2  "idr"
  From4/1 -> Mux2/2  "Tr"
  From1/1 -> Rotor Side/3  "vdr_ref"
  From49/1 -> Mux1/1  "iqr_ref"
  Mux13/1 -> Grid Side/4
  From44/1 -> Mux13/2  "Qg_mes"
  From20/1 -> Mux13/1  "Qg_ref"
  Mux12/1 -> Grid Side/6
  From25/1 -> Mux12/1  "vqg_ref"
  From21/1 -> Mux12/2  "vqg_mes"
  Gain2/1 -> Mux9/4, To Workspace3/1  "P rotor"
  From38/1 -> Gain6/1
  Mux9/1 -> Power/1
  From37/1 -> Mux9/1  "P meca"
  From46/1 -> Gain2/1
  Gain7/1 -> Mux9/3, To Workspace2/1  "P stator"
  From41/1 -> Gain7/1
  Gain6/1 -> Mux9/2, To Workspace1/1  "P out"
  Mux11/1 -> Grid Side/3
  From19/1 -> Mux11/2  "vdg_mes"
  From18/1 -> Mux11/1  "vdg_ref"
  From5/1 -> Mux8/1  "P_mech"
  Constant/1 -> Mux6/3, To Workspace7/1  "synchronous rotor speed"
  From47/1 -> Mux6/2  "omega_m"
  From22/1 -> External Quantities/1  "Wind speed"
  Mux6/1 -> External Quantities/2
  Mux8/1 -> External Quantities/3
  From26/1 -> Gain/1
  Gain/1 -> Mux8/2  "P_grid"
  From2/1 -> Rotor Side/1  "omega_m"
  From13/1 -> Mux5/1  "idg_ref"
  Mux4/1 -> Grid Side/1
  From14/1 -> Mux5/2  "idg_mes"
  Mux5/1 -> Grid Side/2
  From8/1 -> Mux4/2  "Vbus_mes"
  Mux7/1 -> Grid Side/5
  From7/1 -> Mux4/1  "Vbus_ref"
  From24/1 -> Mux7/2  "iqg_mes"
  From17/1 -> Grid Side/9  "Ir"
  From15/1 -> Grid Side/7  "Vout"
  From23/1 -> Mux7/1  "iqg_ref"
  From16/1 -> Grid Side/8  "Ig"
  From12/1 -> Rotor Side/9  "Ir"
  From11/1 -> Rotor Side/8  "Iout"
  From10/1 -> Rotor Side/7  "Vout"
  From9/1 -> Rotor Side/6  "vqr_ref"
  From31/1 -> Mux3/1  "idr_ref"
  Mux3/1 -> Rotor Side/5
  Mux2/1 -> Rotor Side/4
  From3/1 -> Mux2/1  "Tr_ref_MPPT"
  Mux1/1 -> Rotor Side/2
  From45/1 -> Mux1/2  "iqr"

== system Complete_Model/Equivalent Wind Park/300MW Eq WT9/Aerodynamic Model ==
  note: Gearbox ratio
  note: Motor convention : Generated power
      |  is negative
  note: \Omega_m blades
  note: Cp
  note: \lambda = \Omega_m R / v_{wind}
  note: C_p (\lambda)
  note: v_{wind}
  Vw : Inport
  C_p (\lambda) : Lookup_n-D  NumberOfTableDimensions=1  BreakpointsForDimension1=tab_lambda  Table=Cp  InternalRulePriority=Speed  RndMeth=Simplest
  Constant : Constant  Value=Radius
  Divide : Product  Inputs=*/
  Divide1 : Product  Inputs=**/
  Fcn2 : Fcn  Expr=0.5*rho*pi*Radius^2*u(2)^3*u(1)
  Gain : Gain  Gain=1/N
  Gain2 : Gain  Gain=n_wt_agg
  Gain4 : Gain  Gain=-1
  Mux2 : Mux  Inputs=2
  Tm : Outport
  tag scope: P_m7=global, Tm7=global, lambda7=global
  wires:
  Gain4/1 -> Divide/1
  Constant/1 -> Divide1/1
  Divide/1 -> Tm/1, [Tm7]
  [omega_m7] -> Divide/2
  Gain2/1 -> Gain4/1, [P_m7]
  Fcn2/1 -> Gain2/1
  Mux2/1 -> Fcn2/1
  C_p (\lambda)/1 -> Mux2/1
  Divide1/1 -> [lambda7], C_p (\lambda)/1  "lambda"
  Vw/1 -> Mux2/2, Divide1/3
  Gain/1 -> Divide1/2
  [omega_m7] -> Gain/1

== system Complete_Model/Equivalent Wind Park/300MW Eq WT9/Grid Side Control ==
  Vbus_ref : Inport
  Qg_ref : Inport  Port=2
  Vbus : Inport  Port=3
  Ig_mes : Inport  Port=4
  Vs_mes : Inport  Port=5
  Demux : Demux  Outputs=2
  Demux1 : Demux  Outputs=2
  Gain : Gain  Gain=Kqg
  Gain1 : Gain  Gain=Kpg
  Normalized for PWM : Gain  Gain=1/(Vbus/2)
  "PI " : Subsystem
  PI id : Subsystem
  PI iq : Subsystem
  Sum : Sum  Inputs=|++
  Vs_mes1 : InportShadow  Port=5
  abc->alpha/beta : Subsystem
  abc->alpha/beta1 : Subsystem
  alpha/beta->abc : Subsystem
  alpha/beta->d/q : Subsystem
  alpha/beta->d/q1 : Subsystem
  angle2 : Subsystem
  cancellation of coupling terms : Subsystem
  d/q->alpha/beta : Subsystem
  Vabc_ref : Outport
  tag scope: Qg_ref7=global, Vbus_ref7=global, idg7=global, idg_ref7=global, iqg7=global, iqg_ref7=global, vdg7=global, vqg7=global
  wires:
  Vs_mes1/1 -> Sum/2
  angle2/1(theta_g) -> [theta_grid7]
  [theta_grid7] -> alpha/beta->d/q1/2(theta)
  [theta_grid7] -> d/q->alpha/beta/2(theta)
  [theta_grid7] -> alpha/beta->d/q/2(theta)
  Demux/2 -> PI iq/2(meas), [iqg7]
  Demux/1 -> PI id/2(meas), [idg7]
  Vbus/1 -> "PI "/2(meas)
  Demux1/1 -> [vdg7]
  Demux1/2 -> [vqg7]
  alpha/beta->d/q1/1(d/q) -> Demux1/1
  abc->alpha/beta1/1(alpha/beta) -> alpha/beta->d/q1/1(D/Q)
  [Vg7] -> abc->alpha/beta1/1(abc)
  cancellation of coupling terms/1(out) -> d/q->alpha/beta/1(d/q)
  Sum/1 -> Normalized for PWM/1
  alpha/beta->abc/1(abc) -> Sum/1
  Qg_ref/1 -> Gain/1, [Qg_ref7]
  Gain1/1 -> PI id/1(ref), [idg_ref7]  "idg"
  "PI "/1(out) -> Gain1/1  "Pg_ref"
  Gain/1 -> [iqg_ref7], PI iq/1(ref)  "iqg"
  Vbus_ref/1 -> "PI "/1(ref), [Vbus_ref7]
  alpha/beta->d/q/1(d/q) -> Demux/1
  PI iq/1(out) -> cancellation of coupling terms/2(Vq*)
  PI id/1(out) -> cancellation of coupling terms/1(Vd*)
  Vs_mes/1 -> angle2/1(Vs_mes)
  Normalized for PWM/1 -> Vabc_ref/1
  abc->alpha/beta/1(alpha/beta) -> alpha/beta->d/q/1(D/Q)
  Ig_mes/1 -> abc->alpha/beta/1(abc)
  d/q->alpha/beta/1(D/Q) -> alpha/beta->abc/1(D/Q)

== system Complete_Model/Equivalent Wind Park/300MW Eq WT9/Power Calculations ==
  Demux{,1..3} (4 alike) : Demux  Outputs=2
  Divide : Product  Inputs=**
  Gain : Gain  Gain=3/2
  Gain1 : Gain  Gain=-1
  Gain2 : Gain  Gain=-1
  Power (3ph, Instantaneous){1..4} (4 alike) : spsPower3phInstantaneousLib/Power (3ph, Instantaneous)
  Scope{1..8,10..13} (12 alike) : Scope
  Scope9 : Scope  NumInputPorts=2
  "Second-Order " : spsSecondOrderFilterLib/Second-Order Filter  FilterType=Lowpass  Fo=17  Zeta=0.7  Ts=7.4074e-05  Initialize=off  Vac_Init="[0,  0,  60]"  Vdc_Init=0  PlotResponse=off  FreqRange=[0, 500, 1]
      settings: AttributesFormatString=f0=%<Fo>
  Second-Order 1 : spsSecondOrderFilterLib/Second-Order Filter  FilterType=Lowpass  Fo=17  Zeta=0.7  Ts=7.4074e-05  Initialize=off  Vac_Init="[0,  0,  60]"  Vdc_Init=0  PlotResponse=off  FreqRange=[0, 500, 1]
      settings: AttributesFormatString=f0=%<Fo>
  Second-Order Low-Pass Filter {1..7} (7 alike) : spsSecondOrderFilterLib/Second-Order Filter  FilterType=Lowpass  Fo=17  Zeta=0.7  Ts=7.4074e-05  Initialize=off  Vac_Init="[0,  0,  60]"  Vdc_Init=0  PlotResponse=off  FreqRange=[0, 500, 1]
      settings: AttributesFormatString=f0=%<Fo>
  Subsystem : Subsystem
  Subsystem1 : Subsystem
  Subsystem2 : Subsystem
  Subsystem3 : Subsystem
  To Workspace1 : ToWorkspace  VariableName=Iout_d7  SaveFormat=Timeseries  SampleTime=-1
  To Workspace2 : ToWorkspace  VariableName=Ig_d7  SaveFormat=Timeseries  SampleTime=-1
  To Workspace21 : ToWorkspace  VariableName=Vout_d7  SaveFormat=Timeseries  SampleTime=-1
  To Workspace3 : ToWorkspace  VariableName=Vg_d7  SaveFormat=Timeseries  SampleTime=-1
  ToFile_Ig_d7 : ToFile  Filename=Ig_d7.mat  SaveFormat=Timeseries
  ToFile_Iout_d7 : ToFile  Filename=Iout_d7.mat  SaveFormat=Timeseries
  ToFile_Vg_d7 : ToFile  Filename=Vg_d7.mat  SaveFormat=Timeseries
  ToFile_Vout_d7 : ToFile  Filename=Vout_d7.mat  SaveFormat=Timeseries
  alpha/beta->d/q : Subsystem
  alpha/beta->d/q1 : Subsystem
  alpha/beta->d/q2 : Subsystem
  alpha/beta->d/q3 : Subsystem
  angle1 : Subsystem
  angle2 : Subsystem
  tag scope: Pg_mes7=global, Pout7=global, Pr_mes_machine7=global, Ps_mes_machine7=global, Qg_mes7=global, Qout7=global, Qr_mes_machine7=global, Qs_mes_machine7=global
  wires:
  [Vg7] -> angle1/1(Vs_mes), Subsystem2/1(abc)
  Subsystem2/1(alpha/beta) -> alpha/beta->d/q2/1(D/Q)
  alpha/beta->d/q2/1(d/q) -> Demux2/1
  Demux2/1 -> To Workspace3/1, Scope10/1, ToFile_Vg_d7/1  "Vg_d7"
  Demux2/2 -> Scope11/1
  Demux3/2 -> Scope13/1
  Demux3/1 -> Scope12/1, To Workspace2/1, ToFile_Ig_d7/1  "Ig_d7"
  alpha/beta->d/q3/1(d/q) -> Demux3/1
  Subsystem3/1(alpha/beta) -> alpha/beta->d/q3/1(D/Q)
  angle1/1(theta_g) -> alpha/beta->d/q3/2(theta), alpha/beta->d/q2/2(theta)
  [Ig7] -> Subsystem3/1(abc)
  Gain2/1 -> Second-Order 1/1
  Gain/1 -> Gain2/1
  Gain1/1 -> Scope9/2
  Second-Order 1/1 -> Scope9/1  "Pout"
  Divide/1 -> Gain/1
  [Iout7] -> Subsystem1/1(abc)
  angle2/1(theta_g) -> alpha/beta->d/q/2(theta), alpha/beta->d/q1/2(theta)
  Subsystem1/1(alpha/beta) -> alpha/beta->d/q1/1(D/Q)
  alpha/beta->d/q1/1(d/q) -> Demux1/1
  Demux1/1 -> To Workspace1/1, Scope7/1, Divide/2, ToFile_Iout_d7/1  "Iout_d7"
  Demux1/2 -> Scope8/1
  Demux/2 -> Scope6/1
  Demux/1 -> Scope2/1, To Workspace21/1, Divide/1, ToFile_Vout_d7/1  "Vout_d7"
  alpha/beta->d/q/1(d/q) -> Demux/1
  Subsystem/1(alpha/beta) -> alpha/beta->d/q/1(D/Q)
  [Vout7] -> Subsystem/1(abc), angle2/1(Vs_mes)
  [Vr_mes7] -> "Power (3ph, Instantaneous)4"/1
  "Power (3ph, Instantaneous)1"/1 -> Second-Order Low-Pass Filter 7/1  "Pr"
  "Power (3ph, Instantaneous)4"/1 -> Second-Order Low-Pass Filter 5/1  "Pr"
  [Vout7] -> "Power (3ph, Instantaneous)2"/1
  "Power (3ph, Instantaneous)3"/2 -> Second-Order Low-Pass Filter 2/1
  [Vg7] -> "Power (3ph, Instantaneous)1"/1
  Second-Order Low-Pass Filter 2/1 -> [Qs_mes_machine7]  "Q_stator"
  [Vs_mes_machine7] -> "Power (3ph, Instantaneous)3"/1
  "Power (3ph, Instantaneous)2"/1 -> "Second-Order "/1
  Second-Order Low-Pass Filter 5/1 -> [Pr_mes_machine7]  "P_rotor"
  [Is_mes_machine7] -> "Power (3ph, Instantaneous)3"/2, Scope4/1
  Second-Order Low-Pass Filter 7/1 -> [Pg_mes7]  "P_rotor"
  [Ir_mes7] -> "Power (3ph, Instantaneous)4"/2, Scope5/1
  "Second-Order "/1 -> [Pout7], Gain1/1  "Pout"
  Second-Order Low-Pass Filter 6/1 -> [Qg_mes7]  "Q_rotor"
  Second-Order Low-Pass Filter 4/1 -> [Qr_mes_machine7]  "Q_rotor"
  "Power (3ph, Instantaneous)3"/1 -> Second-Order Low-Pass Filter 3/1  "Ps"
  [Ig7] -> Scope1/1, "Power (3ph, Instantaneous)1"/2
  "Power (3ph, Instantaneous)4"/2 -> Second-Order Low-Pass Filter 4/1
  [Iout7] -> "Power (3ph, Instantaneous)2"/2, Scope3/1
  "Power (3ph, Instantaneous)1"/2 -> Second-Order Low-Pass Filter 6/1
  Second-Order Low-Pass Filter 3/1 -> [Ps_mes_machine7]  "P_stator"
  Second-Order Low-Pass Filter 1/1 -> [Qout7]  "Qout"
  "Power (3ph, Instantaneous)2"/2 -> Second-Order Low-Pass Filter 1/1

== system Complete_Model/Equivalent Wind Park/300MW Eq WT9/Rotor Side Control ==
  note: Stator referred
  idr_ref : Inport
  Ir_mes : Inport  Port=2
  Vs_mes : Inport  Port=3
  theta_m_mes : Inport  Port=4
  speed_mes : Inport  Port=5
  D/Q->abc : Subsystem
  D/Q->d/q : Subsystem
  Demux : Demux  Outputs=2
  Gain : Gain  Gain=1/(-1.5*p*(Lm/Ls)*Fs)
  Gain1 : Gain  Gain=1/u
  Gain2 : Gain  Gain=1/u
  MPPT : Subsystem
  Normalized for PWM : Gain  Gain=1/(Vbus/2)
  PI id : Subsystem
  PI iq : Subsystem
  abc->alpha/beta : Subsystem
  angle : Subsystem
  cancellation of coupling terms : Subsystem
  d/q->D/Q : Subsystem
  Vabc_ref : Outport
  tag scope: Tr_ref7=global, idr7=global, idr_ref7=global, iqr7=global, iqr_ref7=global, vrabc_ref7=global
  wires:
  MPPT/1(Tm,opt) -> Gain/1, [Tr_ref7]
  d/q->D/Q/1(D/Q) -> D/Q->abc/1(D/Q)
  abc->alpha/beta/1(alpha/beta) -> D/Q->d/q/1(D/Q)
  Gain2/1 -> [vrabc_ref7], Normalized for PWM/1  "Rotor referred"
  D/Q->abc/1(abc) -> Gain2/1
  Normalized for PWM/1 -> Vabc_ref/1
  Gain1/1 -> abc->alpha/beta/1(abc)
  idr_ref/1 -> PI id/1(ref), [idr_ref7]
  Gain/1 -> PI iq/1(ref), [iqr_ref7]
  Demux/2 -> PI iq/2(meas), [iqr7]
  Demux/1 -> [idr7], PI id/2(meas)
  D/Q->d/q/1(d/q) -> Demux/1  "i_dq = rotor ref frame"
  speed_mes/1 -> MPPT/1(omega_m), cancellation of coupling terms/3(omega_m)
  cancellation of coupling terms/1(out) -> d/q->D/Q/1(d/q)  "Vdr_ref,Vqr_ref"
  PI iq/1(out) -> cancellation of coupling terms/2(Vq*)
  PI id/1(out) -> cancellation of coupling terms/1(Vd*)
  angle/1(theta_r) -> D/Q->d/q/2(theta), d/q->D/Q/2(theta)
  Vs_mes/1 -> angle/1(Vs_mes)
  theta_m_mes/1 -> angle/2(theta_m)
  Ir_mes/1 -> Gain1/1

== system Complete_Model/Equivalent Wind Park/300MW Eq WT9/Subsystem ==
  In1 : Inport
  Bus Selector : BusSelector  OutputSignals=Mechanical.Rotor angle thetam (rad),Mechanical.Rotor speed (wm),Mechanical.Electromagnetic torque Te (N*m)
  Second-Order Low-Pass Filter 3 : spsSecondOrderFilterLib/Second-Order Filter  FilterType=Lowpass  Fo=17  Zeta=0.7  Ts=7.4074e-05  Initialize=off  Vac_Init="[0,  0,  60]"  Vdc_Init=0  PlotResponse=off  FreqRange=[0, 500, 1]
      settings: AttributesFormatString=f0=%<Fo>
  tag scope: Tr7=global, omega_m7=global, theta7=global
  wires:
  Second-Order Low-Pass Filter 3/1 -> [omega_m7]
  Bus Selector/3 -> [Tr7]  "<Electromagnetic torque Te (N*m)>"
  Bus Selector/1 -> [theta7]  "<Rotor angle thetam (rad)>"
  In1/1 -> Bus Selector/1
  Bus Selector/2 -> Second-Order Low-Pass Filter 3/1  "<Rotor speed (wm)>"

== system Complete_Model/Equivalent Wind Park/300MW Eq WT9/Subsystem1 ==
  Constant4 : Constant  Value=0
  Step : Step  Time=50  After=-0.2*Ps  SampleTime=0
  Sum14 : Sum  Inputs=++|
  Out1 : Outport
  wires:
  Sum14/1 -> Out1/1
  Step/1 -> Sum14/1
  Constant4/1 -> Sum14/2

== system Complete_Model/Equivalent Wind Park/300MW Eq WT9/Subsystem2 ==
  Constant1 : Constant  Value=9
  Ramp1 : simulink/Sources/Ramp  slope=-0.4  start=13  InitialOutput=0
  Ramp2 : simulink/Sources/Ramp  slope=0.4  start=8  InitialOutput=0
  Sum1 : Sum  Inputs=++|
  Sum2 : Sum  Inputs=++|
  v_wind : Outport
  tag scope: v_wind7=global
  wires:
  Ramp1/1 -> Sum1/2
  Constant1/1 -> Sum2/2
  Ramp2/1 -> Sum1/1
  Sum1/1 -> Sum2/1
  Sum2/1 -> [v_wind7], v_wind/1

== system Complete_Model/Equivalent Wind Park/300MW Eq WT9/Subsystem8 ==
  Constant4 : Constant  Value=0
  Step : Step  Time=55  After=Idr_rated*0.2  SampleTime=0
  Sum14 : Sum  Inputs=++|
  Out1 : Outport
  wires:
  Sum14/1 -> Out1/1
  Constant4/1 -> Sum14/2
  Step/1 -> Sum14/1
